simulink model "r2_ratty2_32k_r203"  (R2012b)
settings: Solver=ode45  StartTime=0.0  StopTime=10.0  RelTol=1e-3
undefined symbols (not defined in the file): Down, pfb_coeff_gen_calc, Up
libraries: xbsIndex_r4 x20024, casper_library_misc x89, casper_library_delays x41, xps_library x14, xbsReplacements_r4 x2, simulink x1, casper_library_scopes x1
(part 131 of 207)

== system r2_ratty2_32k_r203/fft/fft_direct/butterfly2_1/twiddle/coeff_gen/cosin ==
  theta : Inport
  misci : Inport  Port=2
  Constant4 : xbsIndex_r4/Constant  const=0  gui_display_data_type=Fixed-point  arith_type=Unsigned  n_bits=1  bin_pt=0  preci_type=Single  exp_width=8  frac_width=24  explicit_period=on  period=1  equ=P=C  opselect=C  inp2=PCIN>>17  opr=+  inp1=P  carry=CIN  dbl_ovrd=off  block_type=constant
  Constant5 : xbsIndex_r4/Constant  const=0  gui_display_data_type=Fixed-point  arith_type=Unsigned  n_bits=1  bin_pt=0  preci_type=Single  exp_width=8  frac_width=24  explicit_period=on  period=1  equ=P=C  opselect=C  inp2=PCIN>>17  opr=+  inp1=P  carry=CIN  dbl_ovrd=off  block_type=constant
  Delay : xbsIndex_r4/Delay  rst=off  infoeditControl=Selection of Reset will increase slice count due to use of real FFs and instead of SRLs  en=off  latency=bram_latency  dbl_ovrd=off  reg_retiming=on  block_type=delay
  Delay10 : xbsIndex_r4/Delay  rst=off  infoeditControl=Selection of Reset will increase slice count due to use of real FFs and instead of SRLs  en=off  latency=bram_latency  dbl_ovrd=off  reg_retiming=on  block_type=delay
  Delay8 : xbsIndex_r4/Delay  rst=off  infoeditControl=Selection of Reset will increase slice count due to use of real FFs and instead of SRLs  en=off  latency=bram_latency  dbl_ovrd=off  reg_retiming=on  block_type=delay
  ROM : xbsIndex_r4/ROM  depth=2^(13)  initVector=[131072 68716199936 68713054208 68709646336 68706500608 68703092736 68699947008 68696539136 68693131264 68689985536 68686577664 68683431936 68680024064 68676878336 68673470464 68670324736 68666916864 68663508992 68660363264 68656955392 68653809664 68650401792 68647256064 68643848192 68640440320 68637294592 68633886720 68630740992 68627333120 68624187393 68620779521 68617371649 68614225921 68610818049 68607672321 68604264449 68601118721 68597710849 68594302977 68591157249 68587749377 68584603649 68581195777 68578050049 68574642177 68571496449 68568088577 68564680705 68561534977 68558127105 68554981378 68551573506 68548427778 68545019906 68541612034 68538466306 68535058434 68531912706 68528504834 68525359106 68521951234 68518543362 68515397634 68511989762 68508844034 68505436163 68502290435 68498882563 68495474691 68492328963 68488921091 68485775363 68482367491 68479221763 68475813891 68472668163 68469260291 68465852420 68462706692 68459298820 68456153092 68452745220 68449599492 68446191620 68442783748 68439638020 68436230148 68433084421 68429676549 68426530821 68423122949 68419715077 68416569349 68413161477 68410015749 68406607877 68403462150 68400054278 68396908550 68393500678 68390092806 68386947078 68383539206 68380393478 68376985607 68373839879 68370432007 68367024135 68363878407 68360470535 68357324807 68353916935 68350771208 68347363336 68343955464 68340809736 68337401864 68334256136 68330848264 68327702537 68324294665 68321148937 68317741065 68314333193 68311187465 68307779593 68304633866 68301225994 68298080266 68294672394 68291264522 68288118794 68284710922 68281565195 68278157323 68275011595 68271603723 68268195851 68265050123 68261642252 68258496524 68255088652 68251942924 68248535052 68245389324 68241981453 68238573581 68235427853 68232019981 68228874253 68225466382 68222320654 68218912782 68215504910 68212359182 68208951310 68205805583 68202397711 68199251983 68195844111 68192436239 68189290512 68185882640 68182736912 68179329040 68176183312 68172775441 68169629713 68166221841 68162813969 68159668241 68156260370 68153114642 68149706770 68146561042 68143153170 68139745299 68136599571 68133191699 68130045971 68126638100 68123492372 68120084500 68116676628 68113530900 68110123029 68106977301 68103569429 68100423701 68097015830 68093870102 68090462230 68087054358 68083908630 68080500759 68077355031 68073947159 68070801431 68067393560 68063985688 68060839960 68057432088 68054286361 68050878489 68047732761 68044324889 68040917018 68037771290 68034363418 68031217690 68027809819 68024664091 68021256219 68018110491 68014702620 68011294748 68008149020 68004741148 68001595421 67998187549 67995041821 67991633949 67988226078 67985080350 67981672478 67978526750 67975118879 67971973151 67968565279 67965419552 67962011680 67958603808 67955458080 67952050209 67948904481 67945496609 67942350882 67938943010 67935535138 67932389410 67928981539 67925835811 67922427939 67919282212 67915874340 67912728612 67909320740 67905912869 67902767141 67899359269 67896213542 67892805670 67889659942 67886252071 67882844199 67879698471 67876290599 67873144872 67869737000 67866591272 67863183401 67860037673 67856629801 67853221930 67850076202 67846668330 67843522603 67840114731 67836969003 67833561132 67830153260 67827007532 67823599661 67820453933 67817046061 67813900334 67810492462 67807346734 67803938863 67800530991 67797385263 67793977392 67790831664 67787423792 67784278065 67780870193 67777462321 67774316594 67770908722 67767762994 67764355123 67761209395 67757801523 67754655796 67751247924 67747840052 67744694325 67741286453 67738140725 67734732854 67731587126 67728179255 67724771383 67721625655 67718217784 67715072056 67711664184 67708518457 67705110585 67701964858 67698556986 67695149114 67692003387 67688595515 67685449787 67682041916 67678896188 67675488317 67672342589 67668934717 67665526846 67662381118 67658973246 67655827519 67652419647 67649273920 67645866048 67642458176 67639312449 67635904577 67632758850 67629350978 67626205250 ...(+94308 chars)]  distributed_mem=Block RAM  rst=off  init_reg=0  en=off  latency=bram_latency  gui_display_data_type=Fixed-point  arith_type=Unsigned  n_bits=n_bits*2  bin_pt=0  preci_type=Single  exp_width=8  frac_width=24  dbl_ovrd=off  optimize=Speed  use_rpm=on  block_type=sprom
  Terminator : Terminator
  Terminator1 : Terminator
  Terminator4 : Terminator
  add_convert0 : Subsystem
  add_convert1 : Subsystem
  assert : xbsIndex_r4/Assert  assert_type=on  type_source=Explicitly  gui_display_data_type=Fixed-point  arith_type=Unsigned  n_bits=13  bin_pt=0  preci_type=Single  exp_width=8  frac_width=24  assert_rate=off  rate_source=Explicitly  period=1  output_port=on  block_type=assert
  c_to_ri : casper_library_misc/c_to_ri  n_bits=n_bits  bin_pt=bin_pt
  invert0 : Subsystem  mask{n_bits=n_bits, bin_pt=bin_pt, neg_latency=neg_latency, mux_latency=mux_latency}
      mask prompts: n_bits "bit width"; bin_pt "binary point"; neg_latency "negate latency"; mux_latency "mux latency"
  invert1 : Subsystem  mask{n_bits=n_bits, bin_pt=bin_pt, neg_latency=neg_latency, mux_latency=mux_latency}
      mask prompts: n_bits "bit width"; bin_pt "binary point"; neg_latency "negate latency"; mux_latency "mux latency"
  -sin : Outport
  -cos : Outport  Port=2
  misco : Outport  Port=3
  wires:
  theta/1 -> assert/1
  assert/1 -> add_convert0/1(theta), add_convert1/1(theta)
  Constant4/1 -> add_convert0/2(misci)
  add_convert0/3(misco) -> Terminator/1
  misci/1 -> add_convert1/2(misci)
  add_convert1/3(misco) -> Delay/1
  add_convert0/2(add) -> ROM/1
  add_convert1/2(add) -> Terminator4/1
  ROM/1 -> c_to_ri/1
  add_convert1/1(negate) -> Delay8/1
  add_convert0/1(negate) -> Delay10/1
  Delay10/1 -> invert0/1(negate)
  Constant5/1 -> invert0/3(misci)
  c_to_ri/1 -> invert0/2(in)
  Delay8/1 -> invert1/1(negate)
  c_to_ri/2 -> invert1/2(in)
  invert0/2(misco) -> Terminator1/1
  Delay/1 -> invert1/3(misci)
  invert0/1(out) -> -sin/1
  invert1/2(misco) -> misco/1
  invert1/1(out) -> -cos/1
